simulink model "v01_aa_rii_16r4t11f_ver120sa"  (Simulink 7.6)
settings: Solver=ode45  StartTime=0.0  StopTime=128000  RelTol=1e-3
undefined symbols (not defined in the file or in its folder): sfp
libraries: xbsIndex_r4 x27287, monroe_library x154, xbsReplacements_r4 x78, casper_library_misc x33, simulink x32, dspsigops x32, xps_library x24
(part 5 of 178)

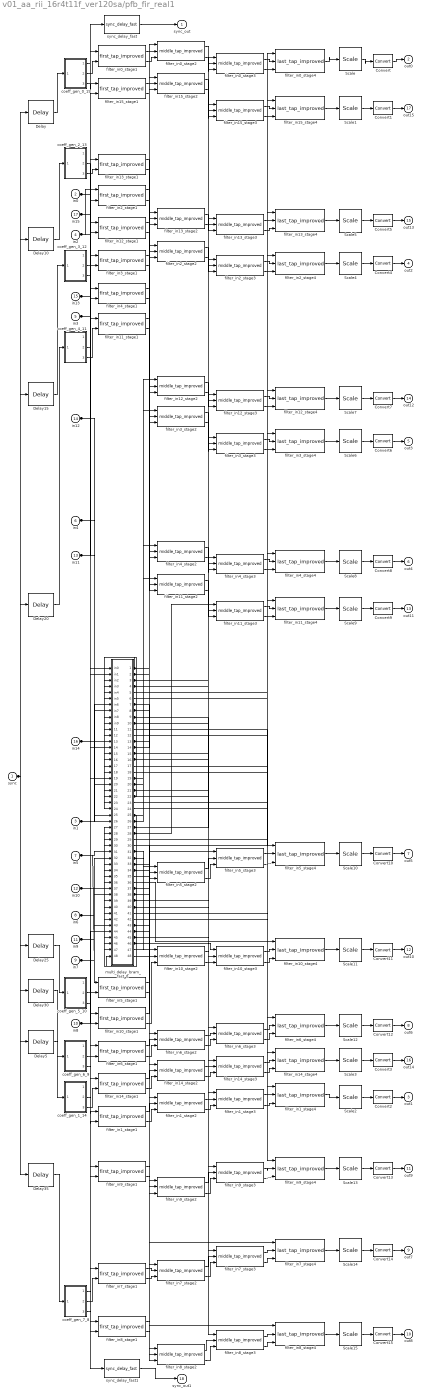
== system v01_aa_rii_16r4t11f_ver120sa/pfb_fir_real1 ==
  sync : Inport
  in0 : Inport  Port=2
  in1 : Inport  Port=3
  in2 : Inport  Port=4
  in3 : Inport  Port=5
  in4 : Inport  Port=6
  in5 : Inport  Port=7
  in6 : Inport  Port=8
  in7 : Inport  Port=9
  in8 : Inport  Port=10
  in9 : Inport  Port=11
  in10 : Inport  Port=12
  in11 : Inport  Port=13
  in12 : Inport  Port=14
  in13 : Inport  Port=15
  in14 : Inport  Port=16
  in15 : Inport  Port=17
  Convert{,1..15} (16 alike) : xbsIndex_r4/Convert  gui_display_data_type=Fixed-point  arith_type="Signed  (2's comp)"  n_bits=18  bin_pt=17  float_type=Single  exp_bits=8  fraction_bits=24  quantization=Truncate  overflow=Wrap  en=off  latency=1  dbl_ovrd=off  pipeline=off  block_type=convert
  Delay{,5,10,15,20,25,30,35} (8 alike) : xbsIndex_r4/Delay  rst=off  infoeditControl=Selection of Reset will increase slice count due to use of real FFs and instead of SRLs  en=off  latency=6  dbl_ovrd=off  reg_retiming=off  block_type=delay
  Scale{,1..15} (16 alike) : xbsIndex_r4/Scale  scale_factor=-1  dbl_ovrd=off  block_type=scale
  coeff_gen_0_15 : Subsystem
  coeff_gen_1_14 : Subsystem
  coeff_gen_2_13 : Subsystem
  coeff_gen_3_12 : Subsystem
  coeff_gen_4_11 : Subsystem
  coeff_gen_5_10 : Subsystem
  coeff_gen_6_9 : Subsystem
  coeff_gen_7_8 : Subsystem
  filter_in0_stage1 : monroe_library/first_tap_improved  SystemSampleTime=-1  data_bin_pt=7  coeff_bit_width=18
  filter_in0_stage2 : monroe_library/middle_tap_improved  SystemSampleTime=-1  data_bin_pt=7  coeff_bit_width=18  stage_num=2
  filter_in0_stage3 : monroe_library/middle_tap_improved  SystemSampleTime=-1  data_bin_pt=7  coeff_bit_width=18  stage_num=3
  filter_in0_stage4 : monroe_library/last_tap_improved  SystemSampleTime=-1  data_bin_pt=7  coeff_bin_pt=17  stage_num=4
  filter_in10_stage1 : monroe_library/first_tap_improved  SystemSampleTime=-1  data_bin_pt=7  coeff_bit_width=18
  filter_in10_stage2 : monroe_library/middle_tap_improved  SystemSampleTime=-1  data_bin_pt=7  coeff_bit_width=18  stage_num=2
  filter_in10_stage3 : monroe_library/middle_tap_improved  SystemSampleTime=-1  data_bin_pt=7  coeff_bit_width=18  stage_num=3
  filter_in10_stage4 : monroe_library/last_tap_improved  SystemSampleTime=-1  data_bin_pt=7  coeff_bin_pt=17  stage_num=4
  filter_in11_stage1 : monroe_library/first_tap_improved  SystemSampleTime=-1  data_bin_pt=7  coeff_bit_width=18
  filter_in11_stage2 : monroe_library/middle_tap_improved  SystemSampleTime=-1  data_bin_pt=7  coeff_bit_width=18  stage_num=2
  filter_in11_stage3 : monroe_library/middle_tap_improved  SystemSampleTime=-1  data_bin_pt=7  coeff_bit_width=18  stage_num=3
  filter_in11_stage4 : monroe_library/last_tap_improved  SystemSampleTime=-1  data_bin_pt=7  coeff_bin_pt=17  stage_num=4
  filter_in12_stage1 : monroe_library/first_tap_improved  SystemSampleTime=-1  data_bin_pt=7  coeff_bit_width=18
  filter_in12_stage2 : monroe_library/middle_tap_improved  SystemSampleTime=-1  data_bin_pt=7  coeff_bit_width=18  stage_num=2
  filter_in12_stage3 : monroe_library/middle_tap_improved  SystemSampleTime=-1  data_bin_pt=7  coeff_bit_width=18  stage_num=3
  filter_in12_stage4 : monroe_library/last_tap_improved  SystemSampleTime=-1  data_bin_pt=7  coeff_bin_pt=17  stage_num=4
  filter_in13_stage1 : monroe_library/first_tap_improved  SystemSampleTime=-1  data_bin_pt=7  coeff_bit_width=18
  filter_in13_stage2 : monroe_library/middle_tap_improved  SystemSampleTime=-1  data_bin_pt=7  coeff_bit_width=18  stage_num=2
  filter_in13_stage3 : monroe_library/middle_tap_improved  SystemSampleTime=-1  data_bin_pt=7  coeff_bit_width=18  stage_num=3
  filter_in13_stage4 : monroe_library/last_tap_improved  SystemSampleTime=-1  data_bin_pt=7  coeff_bin_pt=17  stage_num=4
  filter_in14_stage1 : monroe_library/first_tap_improved  SystemSampleTime=-1  data_bin_pt=7  coeff_bit_width=18
  filter_in14_stage2 : monroe_library/middle_tap_improved  SystemSampleTime=-1  data_bin_pt=7  coeff_bit_width=18  stage_num=2
  filter_in14_stage3 : monroe_library/middle_tap_improved  SystemSampleTime=-1  data_bin_pt=7  coeff_bit_width=18  stage_num=3
  filter_in14_stage4 : monroe_library/last_tap_improved  SystemSampleTime=-1  data_bin_pt=7  coeff_bin_pt=17  stage_num=4
  filter_in15_stage1 : monroe_library/first_tap_improved  SystemSampleTime=-1  data_bin_pt=7  coeff_bit_width=18
  filter_in15_stage2 : monroe_library/middle_tap_improved  SystemSampleTime=-1  data_bin_pt=7  coeff_bit_width=18  stage_num=2
  filter_in15_stage3 : monroe_library/middle_tap_improved  SystemSampleTime=-1  data_bin_pt=7  coeff_bit_width=18  stage_num=3
  filter_in15_stage4 : monroe_library/last_tap_improved  SystemSampleTime=-1  data_bin_pt=7  coeff_bin_pt=17  stage_num=4
  filter_in1_stage1 : monroe_library/first_tap_improved  SystemSampleTime=-1  data_bin_pt=7  coeff_bit_width=18
  filter_in1_stage2 : monroe_library/middle_tap_improved  SystemSampleTime=-1  data_bin_pt=7  coeff_bit_width=18  stage_num=2
  filter_in1_stage3 : monroe_library/middle_tap_improved  SystemSampleTime=-1  data_bin_pt=7  coeff_bit_width=18  stage_num=3
  filter_in1_stage4 : monroe_library/last_tap_improved  SystemSampleTime=-1  data_bin_pt=7  coeff_bin_pt=17  stage_num=4
  filter_in2_stage1 : monroe_library/first_tap_improved  SystemSampleTime=-1  data_bin_pt=7  coeff_bit_width=18
  filter_in2_stage2 : monroe_library/middle_tap_improved  SystemSampleTime=-1  data_bin_pt=7  coeff_bit_width=18  stage_num=2
  filter_in2_stage3 : monroe_library/middle_tap_improved  SystemSampleTime=-1  data_bin_pt=7  coeff_bit_width=18  stage_num=3
  filter_in2_stage4 : monroe_library/last_tap_improved  SystemSampleTime=-1  data_bin_pt=7  coeff_bin_pt=17  stage_num=4
  filter_in3_stage1 : monroe_library/first_tap_improved  SystemSampleTime=-1  data_bin_pt=7  coeff_bit_width=18
  filter_in3_stage2 : monroe_library/middle_tap_improved  SystemSampleTime=-1  data_bin_pt=7  coeff_bit_width=18  stage_num=2
  filter_in3_stage3 : monroe_library/middle_tap_improved  SystemSampleTime=-1  data_bin_pt=7  coeff_bit_width=18  stage_num=3
  filter_in3_stage4 : monroe_library/last_tap_improved  SystemSampleTime=-1  data_bin_pt=7  coeff_bin_pt=17  stage_num=4
  filter_in4_stage1 : monroe_library/first_tap_improved  SystemSampleTime=-1  data_bin_pt=7  coeff_bit_width=18
  filter_in4_stage2 : monroe_library/middle_tap_improved  SystemSampleTime=-1  data_bin_pt=7  coeff_bit_width=18  stage_num=2
  filter_in4_stage3 : monroe_library/middle_tap_improved  SystemSampleTime=-1  data_bin_pt=7  coeff_bit_width=18  stage_num=3
  filter_in4_stage4 : monroe_library/last_tap_improved  SystemSampleTime=-1  data_bin_pt=7  coeff_bin_pt=17  stage_num=4
  filter_in5_stage1 : monroe_library/first_tap_improved  SystemSampleTime=-1  data_bin_pt=7  coeff_bit_width=18
  filter_in5_stage2 : monroe_library/middle_tap_improved  SystemSampleTime=-1  data_bin_pt=7  coeff_bit_width=18  stage_num=2
  filter_in5_stage3 : monroe_library/middle_tap_improved  SystemSampleTime=-1  data_bin_pt=7  coeff_bit_width=18  stage_num=3
  filter_in5_stage4 : monroe_library/last_tap_improved  SystemSampleTime=-1  data_bin_pt=7  coeff_bin_pt=17  stage_num=4
  filter_in6_stage1 : monroe_library/first_tap_improved  SystemSampleTime=-1  data_bin_pt=7  coeff_bit_width=18
  filter_in6_stage2 : monroe_library/middle_tap_improved  SystemSampleTime=-1  data_bin_pt=7  coeff_bit_width=18  stage_num=2
  filter_in6_stage3 : monroe_library/middle_tap_improved  SystemSampleTime=-1  data_bin_pt=7  coeff_bit_width=18  stage_num=3
  filter_in6_stage4 : monroe_library/last_tap_improved  SystemSampleTime=-1  data_bin_pt=7  coeff_bin_pt=17  stage_num=4
  filter_in7_stage1 : monroe_library/first_tap_improved  SystemSampleTime=-1  data_bin_pt=7  coeff_bit_width=18
  filter_in7_stage2 : monroe_library/middle_tap_improved  SystemSampleTime=-1  data_bin_pt=7  coeff_bit_width=18  stage_num=2
  filter_in7_stage3 : monroe_library/middle_tap_improved  SystemSampleTime=-1  data_bin_pt=7  coeff_bit_width=18  stage_num=3
  filter_in7_stage4 : monroe_library/last_tap_improved  SystemSampleTime=-1  data_bin_pt=7  coeff_bin_pt=17  stage_num=4
  filter_in8_stage1 : monroe_library/first_tap_improved  SystemSampleTime=-1  data_bin_pt=7  coeff_bit_width=18
  filter_in8_stage2 : monroe_library/middle_tap_improved  SystemSampleTime=-1  data_bin_pt=7  coeff_bit_width=18  stage_num=2
  filter_in8_stage3 : monroe_library/middle_tap_improved  SystemSampleTime=-1  data_bin_pt=7  coeff_bit_width=18  stage_num=3
  filter_in8_stage4 : monroe_library/last_tap_improved  SystemSampleTime=-1  data_bin_pt=7  coeff_bin_pt=17  stage_num=4
  filter_in9_stage1 : monroe_library/first_tap_improved  SystemSampleTime=-1  data_bin_pt=7  coeff_bit_width=18
  filter_in9_stage2 : monroe_library/middle_tap_improved  SystemSampleTime=-1  data_bin_pt=7  coeff_bit_width=18  stage_num=2
  filter_in9_stage3 : monroe_library/middle_tap_improved  SystemSampleTime=-1  data_bin_pt=7  coeff_bit_width=18  stage_num=3
  filter_in9_stage4 : monroe_library/last_tap_improved  SystemSampleTime=-1  data_bin_pt=7  coeff_bin_pt=17  stage_num=4
  multi_delay_bram_fast_0 : Subsystem
  sync_delay_fast : monroe_library/sync_delay_fast  SystemSampleTime=-1  delay_len=385
  sync_delay_fast1 : monroe_library/sync_delay_fast  SystemSampleTime=-1  delay_len=385
  sync_out : Outport
  out0 : Outport  Port=2
  out1 : Outport  Port=3
  out2 : Outport  Port=4
  out3 : Outport  Port=5
  out4 : Outport  Port=6
  out5 : Outport  Port=7
  out6 : Outport  Port=8
  out7 : Outport  Port=9
  out8 : Outport  Port=10
  out9 : Outport  Port=11
  out10 : Outport  Port=12
  out11 : Outport  Port=13
  out12 : Outport  Port=14
  out13 : Outport  Port=15
  out14 : Outport  Port=16
  out15 : Outport  Port=17
  sync_out1 : Outport  Port=18
  wires:
  sync/1 -> Delay35/1, Delay30/1, Delay25/1, Delay20/1, Delay15/1, Delay10/1, Delay5/1, Delay/1
  in0/1 -> multi_delay_bram_fast_0/1(in0), filter_in0_stage1/1
  in15/1 -> multi_delay_bram_fast_0/2(in1), filter_in15_stage1/1
  in1/1 -> filter_in1_stage1/1, multi_delay_bram_fast_0/7(in6)
  in14/1 -> filter_in14_stage1/1, multi_delay_bram_fast_0/8(in7)
  in2/1 -> filter_in2_stage1/1, multi_delay_bram_fast_0/13(in12)
  in13/1 -> filter_in13_stage1/1, multi_delay_bram_fast_0/14(in13)
  in3/1 -> filter_in3_stage1/1, multi_delay_bram_fast_0/19(in18)
  in12/1 -> filter_in12_stage1/1, multi_delay_bram_fast_0/20(in19)
  in4/1 -> filter_in4_stage1/1, multi_delay_bram_fast_0/25(in24)
  in11/1 -> filter_in11_stage1/1, multi_delay_bram_fast_0/26(in25)
  in5/1 -> filter_in5_stage1/1, multi_delay_bram_fast_0/31(in30)
  in10/1 -> filter_in10_stage1/1, multi_delay_bram_fast_0/32(in31)
  in6/1 -> filter_in6_stage1/1, multi_delay_bram_fast_0/37(in36)
  in9/1 -> filter_in9_stage1/1, multi_delay_bram_fast_0/38(in37)
  in7/1 -> filter_in7_stage1/1, multi_delay_bram_fast_0/43(in42)
  in8/1 -> filter_in8_stage1/1, multi_delay_bram_fast_0/44(in43)
  multi_delay_bram_fast_0/1(out0) -> filter_in0_stage2/1, multi_delay_bram_fast_0/3(in2)
  multi_delay_bram_fast_0/2(out1) -> filter_in15_stage2/1, multi_delay_bram_fast_0/4(in3)
  multi_delay_bram_fast_0/3(out2) -> filter_in0_stage3/1, multi_delay_bram_fast_0/5(in4)
  multi_delay_bram_fast_0/4(out3) -> filter_in15_stage3/1, multi_delay_bram_fast_0/6(in5)
  multi_delay_bram_fast_0/7(out6) -> filter_in1_stage2/1, multi_delay_bram_fast_0/9(in8)
  multi_delay_bram_fast_0/8(out7) -> filter_in14_stage2/1, multi_delay_bram_fast_0/10(in9)
  multi_delay_bram_fast_0/9(out8) -> filter_in1_stage3/1, multi_delay_bram_fast_0/11(in10)
  multi_delay_bram_fast_0/10(out9) -> filter_in14_stage3/1, multi_delay_bram_fast_0/12(in11)
  multi_delay_bram_fast_0/13(out12) -> filter_in2_stage2/1, multi_delay_bram_fast_0/15(in14)
  multi_delay_bram_fast_0/14(out13) -> filter_in13_stage2/1, multi_delay_bram_fast_0/16(in15)
  multi_delay_bram_fast_0/15(out14) -> filter_in2_stage3/1, multi_delay_bram_fast_0/17(in16)
  multi_delay_bram_fast_0/16(out15) -> filter_in13_stage3/1, multi_delay_bram_fast_0/18(in17)
  multi_delay_bram_fast_0/19(out18) -> filter_in3_stage2/1, multi_delay_bram_fast_0/21(in20)
  multi_delay_bram_fast_0/20(out19) -> filter_in12_stage2/1, multi_delay_bram_fast_0/22(in21)
  multi_delay_bram_fast_0/21(out20) -> filter_in3_stage3/1, multi_delay_bram_fast_0/23(in22)
  multi_delay_bram_fast_0/22(out21) -> filter_in12_stage3/1, multi_delay_bram_fast_0/24(in23)
  multi_delay_bram_fast_0/25(out24) -> filter_in4_stage2/1, multi_delay_bram_fast_0/27(in26)
  multi_delay_bram_fast_0/26(out25) -> filter_in11_stage2/1, multi_delay_bram_fast_0/28(in27)
  multi_delay_bram_fast_0/27(out26) -> filter_in4_stage3/1, multi_delay_bram_fast_0/29(in28)
  multi_delay_bram_fast_0/28(out27) -> filter_in11_stage3/1, multi_delay_bram_fast_0/30(in29)
  multi_delay_bram_fast_0/31(out30) -> filter_in5_stage2/1, multi_delay_bram_fast_0/33(in32)
  multi_delay_bram_fast_0/32(out31) -> filter_in10_stage2/1, multi_delay_bram_fast_0/34(in33)
  multi_delay_bram_fast_0/33(out32) -> filter_in5_stage3/1, multi_delay_bram_fast_0/35(in34)
  multi_delay_bram_fast_0/34(out33) -> filter_in10_stage3/1, multi_delay_bram_fast_0/36(in35)
  multi_delay_bram_fast_0/37(out36) -> filter_in6_stage2/1, multi_delay_bram_fast_0/39(in38)
  multi_delay_bram_fast_0/38(out37) -> filter_in9_stage2/1, multi_delay_bram_fast_0/40(in39)
  multi_delay_bram_fast_0/39(out38) -> filter_in6_stage3/1, multi_delay_bram_fast_0/41(in40)
  multi_delay_bram_fast_0/40(out39) -> filter_in9_stage3/1, multi_delay_bram_fast_0/42(in41)
  multi_delay_bram_fast_0/43(out42) -> filter_in7_stage2/1, multi_delay_bram_fast_0/45(in44)
  multi_delay_bram_fast_0/44(out43) -> filter_in8_stage2/1, multi_delay_bram_fast_0/46(in45)
  multi_delay_bram_fast_0/45(out44) -> filter_in7_stage3/1, multi_delay_bram_fast_0/47(in46)
  multi_delay_bram_fast_0/46(out45) -> filter_in8_stage3/1, multi_delay_bram_fast_0/48(in47)
  sync_delay_fast1/1 -> sync_out1/1
  coeff_gen_7_8/1(sync_out) -> sync_delay_fast1/1
  sync_delay_fast/1 -> sync_out/1
  coeff_gen_0_15/1(sync_out) -> sync_delay_fast/1
  Convert15/1 -> out8/1
  Scale15/1 -> Convert15/1
  Convert14/1 -> out7/1
  Scale14/1 -> Convert14/1
  filter_in8_stage4/1 -> Scale15/1
  filter_in7_stage4/1 -> Scale14/1
  filter_in8_stage3/2 -> filter_in8_stage4/3
  filter_in8_stage3/1 -> filter_in8_stage4/2
  multi_delay_bram_fast_0/48(out47) -> filter_in8_stage4/1
  filter_in7_stage3/2 -> filter_in7_stage4/3
  filter_in7_stage3/1 -> filter_in7_stage4/2
  multi_delay_bram_fast_0/47(out46) -> filter_in7_stage4/1
  filter_in8_stage2/2 -> filter_in8_stage3/3
  filter_in8_stage2/1 -> filter_in8_stage3/2
  filter_in7_stage2/2 -> filter_in7_stage3/3
  filter_in7_stage2/1 -> filter_in7_stage3/2
  filter_in8_stage1/2 -> filter_in8_stage2/3
  filter_in8_stage1/1 -> filter_in8_stage2/2
  filter_in7_stage1/2 -> filter_in7_stage2/3
  filter_in7_stage1/1 -> filter_in7_stage2/2
  coeff_gen_7_8/3(coeff_rev) -> filter_in8_stage1/2
  coeff_gen_7_8/2(coeff) -> filter_in7_stage1/2
  Delay35/1 -> coeff_gen_7_8/1(sync)
  Convert13/1 -> out9/1
  Scale13/1 -> Convert13/1
  Convert12/1 -> out6/1
  Scale12/1 -> Convert12/1
  filter_in9_stage4/1 -> Scale13/1
  filter_in6_stage4/1 -> Scale12/1
  filter_in9_stage3/2 -> filter_in9_stage4/3
  filter_in9_stage3/1 -> filter_in9_stage4/2
  multi_delay_bram_fast_0/42(out41) -> filter_in9_stage4/1
  filter_in6_stage3/2 -> filter_in6_stage4/3
  filter_in6_stage3/1 -> filter_in6_stage4/2
  multi_delay_bram_fast_0/41(out40) -> filter_in6_stage4/1
  filter_in9_stage2/2 -> filter_in9_stage3/3
  filter_in9_stage2/1 -> filter_in9_stage3/2
  filter_in6_stage2/2 -> filter_in6_stage3/3
  filter_in6_stage2/1 -> filter_in6_stage3/2
  filter_in9_stage1/2 -> filter_in9_stage2/3
  filter_in9_stage1/1 -> filter_in9_stage2/2
  filter_in6_stage1/2 -> filter_in6_stage2/3
  filter_in6_stage1/1 -> filter_in6_stage2/2
  coeff_gen_6_9/3(coeff_rev) -> filter_in9_stage1/2
  coeff_gen_6_9/2(coeff) -> filter_in6_stage1/2
  Delay30/1 -> coeff_gen_6_9/1(sync)
  Convert11/1 -> out10/1
  Scale11/1 -> Convert11/1
  Convert10/1 -> out5/1
  Scale10/1 -> Convert10/1
  filter_in10_stage4/1 -> Scale11/1
  filter_in5_stage4/1 -> Scale10/1
  filter_in10_stage3/2 -> filter_in10_stage4/3
  filter_in10_stage3/1 -> filter_in10_stage4/2
  multi_delay_bram_fast_0/36(out35) -> filter_in10_stage4/1
  filter_in5_stage3/2 -> filter_in5_stage4/3
  filter_in5_stage3/1 -> filter_in5_stage4/2
  multi_delay_bram_fast_0/35(out34) -> filter_in5_stage4/1
  filter_in10_stage2/2 -> filter_in10_stage3/3
  filter_in10_stage2/1 -> filter_in10_stage3/2
  filter_in5_stage2/2 -> filter_in5_stage3/3
  filter_in5_stage2/1 -> filter_in5_stage3/2
  filter_in10_stage1/2 -> filter_in10_stage2/3
  filter_in10_stage1/1 -> filter_in10_stage2/2
  filter_in5_stage1/2 -> filter_in5_stage2/3
  filter_in5_stage1/1 -> filter_in5_stage2/2
  coeff_gen_5_10/3(coeff_rev) -> filter_in10_stage1/2
  coeff_gen_5_10/2(coeff) -> filter_in5_stage1/2
  Delay25/1 -> coeff_gen_5_10/1(sync)
  Convert9/1 -> out11/1
  Scale9/1 -> Convert9/1
  Convert8/1 -> out4/1
  Scale8/1 -> Convert8/1
  filter_in11_stage4/1 -> Scale9/1
  filter_in4_stage4/1 -> Scale8/1
  filter_in11_stage3/2 -> filter_in11_stage4/3
  filter_in11_stage3/1 -> filter_in11_stage4/2
  multi_delay_bram_fast_0/30(out29) -> filter_in11_stage4/1
  filter_in4_stage3/2 -> filter_in4_stage4/3
  filter_in4_stage3/1 -> filter_in4_stage4/2
  multi_delay_bram_fast_0/29(out28) -> filter_in4_stage4/1
  filter_in11_stage2/2 -> filter_in11_stage3/3
  filter_in11_stage2/1 -> filter_in11_stage3/2
  filter_in4_stage2/2 -> filter_in4_stage3/3
  filter_in4_stage2/1 -> filter_in4_stage3/2
  filter_in11_stage1/2 -> filter_in11_stage2/3
  filter_in11_stage1/1 -> filter_in11_stage2/2
  filter_in4_stage1/2 -> filter_in4_stage2/3
  filter_in4_stage1/1 -> filter_in4_stage2/2
  coeff_gen_4_11/3(coeff_rev) -> filter_in11_stage1/2
  coeff_gen_4_11/2(coeff) -> filter_in4_stage1/2
  Delay20/1 -> coeff_gen_4_11/1(sync)
  Convert7/1 -> out12/1
  Scale7/1 -> Convert7/1
  Convert6/1 -> out3/1
  Scale6/1 -> Convert6/1
  filter_in12_stage4/1 -> Scale7/1
  filter_in3_stage4/1 -> Scale6/1
  filter_in12_stage3/2 -> filter_in12_stage4/3
  filter_in12_stage3/1 -> filter_in12_stage4/2
  multi_delay_bram_fast_0/24(out23) -> filter_in12_stage4/1
  filter_in3_stage3/2 -> filter_in3_stage4/3
  filter_in3_stage3/1 -> filter_in3_stage4/2
  multi_delay_bram_fast_0/23(out22) -> filter_in3_stage4/1
  filter_in12_stage2/2 -> filter_in12_stage3/3
  filter_in12_stage2/1 -> filter_in12_stage3/2
  filter_in3_stage2/2 -> filter_in3_stage3/3
  filter_in3_stage2/1 -> filter_in3_stage3/2
  filter_in12_stage1/2 -> filter_in12_stage2/3
  filter_in12_stage1/1 -> filter_in12_stage2/2
  filter_in3_stage1/2 -> filter_in3_stage2/3
  filter_in3_stage1/1 -> filter_in3_stage2/2
  coeff_gen_3_12/3(coeff_rev) -> filter_in12_stage1/2
  coeff_gen_3_12/2(coeff) -> filter_in3_stage1/2
  Delay15/1 -> coeff_gen_3_12/1(sync)
  Convert5/1 -> out13/1
  Scale5/1 -> Convert5/1
  Convert4/1 -> out2/1
  Scale4/1 -> Convert4/1
  filter_in13_stage4/1 -> Scale5/1
  filter_in2_stage4/1 -> Scale4/1
  filter_in13_stage3/2 -> filter_in13_stage4/3
  filter_in13_stage3/1 -> filter_in13_stage4/2
  multi_delay_bram_fast_0/18(out17) -> filter_in13_stage4/1
  filter_in2_stage3/2 -> filter_in2_stage4/3
  filter_in2_stage3/1 -> filter_in2_stage4/2
  multi_delay_bram_fast_0/17(out16) -> filter_in2_stage4/1
  filter_in13_stage2/2 -> filter_in13_stage3/3
  filter_in13_stage2/1 -> filter_in13_stage3/2
  filter_in2_stage2/2 -> filter_in2_stage3/3
  filter_in2_stage2/1 -> filter_in2_stage3/2
  filter_in13_stage1/2 -> filter_in13_stage2/3
  filter_in13_stage1/1 -> filter_in13_stage2/2
  filter_in2_stage1/2 -> filter_in2_stage2/3
  filter_in2_stage1/1 -> filter_in2_stage2/2
  coeff_gen_2_13/3(coeff_rev) -> filter_in13_stage1/2
  coeff_gen_2_13/2(coeff) -> filter_in2_stage1/2
  Delay10/1 -> coeff_gen_2_13/1(sync)
  Convert3/1 -> out14/1
  Scale3/1 -> Convert3/1
  Convert2/1 -> out1/1
  Scale2/1 -> Convert2/1
  filter_in14_stage4/1 -> Scale3/1
  filter_in1_stage4/1 -> Scale2/1
  filter_in14_stage3/2 -> filter_in14_stage4/3
  filter_in14_stage3/1 -> filter_in14_stage4/2
  multi_delay_bram_fast_0/12(out11) -> filter_in14_stage4/1
  filter_in1_stage3/2 -> filter_in1_stage4/3
  filter_in1_stage3/1 -> filter_in1_stage4/2
  multi_delay_bram_fast_0/11(out10) -> filter_in1_stage4/1
  filter_in14_stage2/2 -> filter_in14_stage3/3
  filter_in14_stage2/1 -> filter_in14_stage3/2
  filter_in1_stage2/2 -> filter_in1_stage3/3
  filter_in1_stage2/1 -> filter_in1_stage3/2
  filter_in14_stage1/2 -> filter_in14_stage2/3
  filter_in14_stage1/1 -> filter_in14_stage2/2
  filter_in1_stage1/2 -> filter_in1_stage2/3
  filter_in1_stage1/1 -> filter_in1_stage2/2
  coeff_gen_1_14/3(coeff_rev) -> filter_in14_stage1/2
  coeff_gen_1_14/2(coeff) -> filter_in1_stage1/2
  Delay5/1 -> coeff_gen_1_14/1(sync)
  Convert1/1 -> out15/1
  Scale1/1 -> Convert1/1
  Convert/1 -> out0/1
  Scale/1 -> Convert/1
  filter_in15_stage4/1 -> Scale1/1
  filter_in0_stage4/1 -> Scale/1
  filter_in15_stage3/2 -> filter_in15_stage4/3
  filter_in15_stage3/1 -> filter_in15_stage4/2
  multi_delay_bram_fast_0/6(out5) -> filter_in15_stage4/1
  filter_in0_stage3/2 -> filter_in0_stage4/3
  filter_in0_stage3/1 -> filter_in0_stage4/2
  multi_delay_bram_fast_0/5(out4) -> filter_in0_stage4/1
  filter_in15_stage2/2 -> filter_in15_stage3/3
  filter_in15_stage2/1 -> filter_in15_stage3/2
  filter_in0_stage2/2 -> filter_in0_stage3/3
  filter_in0_stage2/1 -> filter_in0_stage3/2
  filter_in15_stage1/2 -> filter_in15_stage2/3
  filter_in15_stage1/1 -> filter_in15_stage2/2
  filter_in0_stage1/2 -> filter_in0_stage2/3
  filter_in0_stage1/1 -> filter_in0_stage2/2
  coeff_gen_0_15/3(coeff_rev) -> filter_in15_stage1/2
  coeff_gen_0_15/2(coeff) -> filter_in0_stage1/2
  Delay/1 -> coeff_gen_0_15/1(sync)

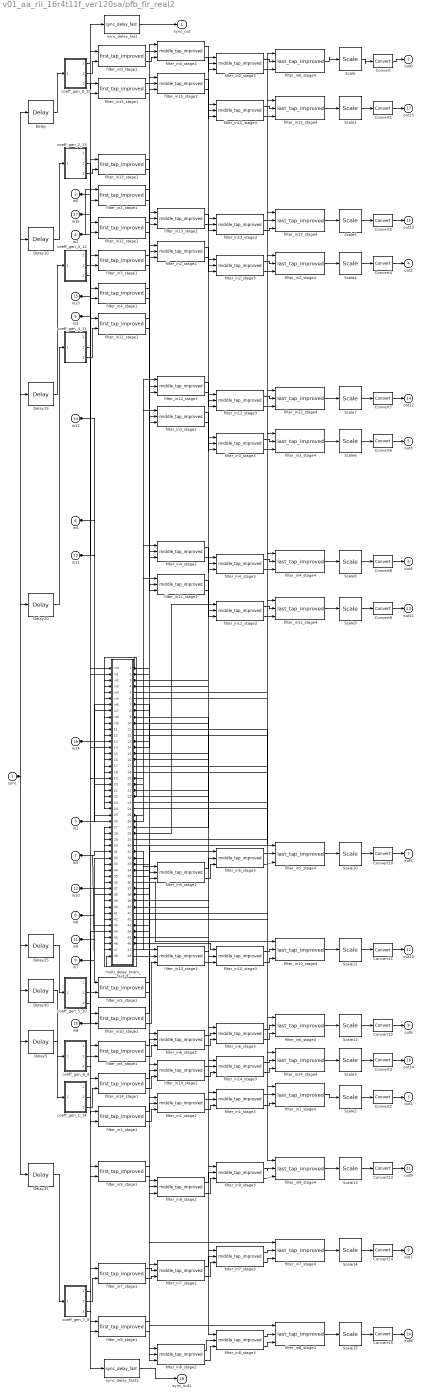
== system v01_aa_rii_16r4t11f_ver120sa/pfb_fir_real2 ==
  sync : Inport
  in0 : Inport  Port=2
  in1 : Inport  Port=3
  in2 : Inport  Port=4
  in3 : Inport  Port=5
  in4 : Inport  Port=6
  in5 : Inport  Port=7
  in6 : Inport  Port=8
  in7 : Inport  Port=9
  in8 : Inport  Port=10
  in9 : Inport  Port=11
  in10 : Inport  Port=12
  in11 : Inport  Port=13
  in12 : Inport  Port=14
  in13 : Inport  Port=15
  in14 : Inport  Port=16
  in15 : Inport  Port=17
  Convert{,1..15} (16 alike) : xbsIndex_r4/Convert  gui_display_data_type=Fixed-point  arith_type="Signed  (2's comp)"  n_bits=18  bin_pt=17  float_type=Single  exp_bits=8  fraction_bits=24  quantization=Truncate  overflow=Wrap  en=off  latency=1  dbl_ovrd=off  pipeline=off  block_type=convert
  Delay{,5,10,15,20,25,30,35} (8 alike) : xbsIndex_r4/Delay  rst=off  infoeditControl=Selection of Reset will increase slice count due to use of real FFs and instead of SRLs  en=off  latency=6  dbl_ovrd=off  reg_retiming=off  block_type=delay
  Scale{,1..15} (16 alike) : xbsIndex_r4/Scale  scale_factor=-1  dbl_ovrd=off  block_type=scale
  coeff_gen_0_15 : Subsystem
  coeff_gen_1_14 : Subsystem
  coeff_gen_2_13 : Subsystem
  coeff_gen_3_12 : Subsystem
  coeff_gen_4_11 : Subsystem
  coeff_gen_5_10 : Subsystem
  coeff_gen_6_9 : Subsystem
  coeff_gen_7_8 : Subsystem
  filter_in0_stage1 : monroe_library/first_tap_improved  SystemSampleTime=-1  data_bin_pt=7  coeff_bit_width=18
  filter_in0_stage2 : monroe_library/middle_tap_improved  SystemSampleTime=-1  data_bin_pt=7  coeff_bit_width=18  stage_num=2
  filter_in0_stage3 : monroe_library/middle_tap_improved  SystemSampleTime=-1  data_bin_pt=7  coeff_bit_width=18  stage_num=3
  filter_in0_stage4 : monroe_library/last_tap_improved  SystemSampleTime=-1  data_bin_pt=7  coeff_bin_pt=17  stage_num=4
  filter_in10_stage1 : monroe_library/first_tap_improved  SystemSampleTime=-1  data_bin_pt=7  coeff_bit_width=18
  filter_in10_stage2 : monroe_library/middle_tap_improved  SystemSampleTime=-1  data_bin_pt=7  coeff_bit_width=18  stage_num=2
  filter_in10_stage3 : monroe_library/middle_tap_improved  SystemSampleTime=-1  data_bin_pt=7  coeff_bit_width=18  stage_num=3
  filter_in10_stage4 : monroe_library/last_tap_improved  SystemSampleTime=-1  data_bin_pt=7  coeff_bin_pt=17  stage_num=4
  filter_in11_stage1 : monroe_library/first_tap_improved  SystemSampleTime=-1  data_bin_pt=7  coeff_bit_width=18
  filter_in11_stage2 : monroe_library/middle_tap_improved  SystemSampleTime=-1  data_bin_pt=7  coeff_bit_width=18  stage_num=2
  filter_in11_stage3 : monroe_library/middle_tap_improved  SystemSampleTime=-1  data_bin_pt=7  coeff_bit_width=18  stage_num=3
  filter_in11_stage4 : monroe_library/last_tap_improved  SystemSampleTime=-1  data_bin_pt=7  coeff_bin_pt=17  stage_num=4
  filter_in12_stage1 : monroe_library/first_tap_improved  SystemSampleTime=-1  data_bin_pt=7  coeff_bit_width=18
  filter_in12_stage2 : monroe_library/middle_tap_improved  SystemSampleTime=-1  data_bin_pt=7  coeff_bit_width=18  stage_num=2
  filter_in12_stage3 : monroe_library/middle_tap_improved  SystemSampleTime=-1  data_bin_pt=7  coeff_bit_width=18  stage_num=3
  filter_in12_stage4 : monroe_library/last_tap_improved  SystemSampleTime=-1  data_bin_pt=7  coeff_bin_pt=17  stage_num=4
  filter_in13_stage1 : monroe_library/first_tap_improved  SystemSampleTime=-1  data_bin_pt=7  coeff_bit_width=18
  filter_in13_stage2 : monroe_library/middle_tap_improved  SystemSampleTime=-1  data_bin_pt=7  coeff_bit_width=18  stage_num=2
  filter_in13_stage3 : monroe_library/middle_tap_improved  SystemSampleTime=-1  data_bin_pt=7  coeff_bit_width=18  stage_num=3
  filter_in13_stage4 : monroe_library/last_tap_improved  SystemSampleTime=-1  data_bin_pt=7  coeff_bin_pt=17  stage_num=4
  filter_in14_stage1 : monroe_library/first_tap_improved  SystemSampleTime=-1  data_bin_pt=7  coeff_bit_width=18
  filter_in14_stage2 : monroe_library/middle_tap_improved  SystemSampleTime=-1  data_bin_pt=7  coeff_bit_width=18  stage_num=2
  filter_in14_stage3 : monroe_library/middle_tap_improved  SystemSampleTime=-1  data_bin_pt=7  coeff_bit_width=18  stage_num=3
  filter_in14_stage4 : monroe_library/last_tap_improved  SystemSampleTime=-1  data_bin_pt=7  coeff_bin_pt=17  stage_num=4
  filter_in15_stage1 : monroe_library/first_tap_improved  SystemSampleTime=-1  data_bin_pt=7  coeff_bit_width=18
  filter_in15_stage2 : monroe_library/middle_tap_improved  SystemSampleTime=-1  data_bin_pt=7  coeff_bit_width=18  stage_num=2
  filter_in15_stage3 : monroe_library/middle_tap_improved  SystemSampleTime=-1  data_bin_pt=7  coeff_bit_width=18  stage_num=3
  filter_in15_stage4 : monroe_library/last_tap_improved  SystemSampleTime=-1  data_bin_pt=7  coeff_bin_pt=17  stage_num=4
  filter_in1_stage1 : monroe_library/first_tap_improved  SystemSampleTime=-1  data_bin_pt=7  coeff_bit_width=18
  filter_in1_stage2 : monroe_library/middle_tap_improved  SystemSampleTime=-1  data_bin_pt=7  coeff_bit_width=18  stage_num=2
  filter_in1_stage3 : monroe_library/middle_tap_improved  SystemSampleTime=-1  data_bin_pt=7  coeff_bit_width=18  stage_num=3
  filter_in1_stage4 : monroe_library/last_tap_improved  SystemSampleTime=-1  data_bin_pt=7  coeff_bin_pt=17  stage_num=4
  filter_in2_stage1 : monroe_library/first_tap_improved  SystemSampleTime=-1  data_bin_pt=7  coeff_bit_width=18
  filter_in2_stage2 : monroe_library/middle_tap_improved  SystemSampleTime=-1  data_bin_pt=7  coeff_bit_width=18  stage_num=2
  filter_in2_stage3 : monroe_library/middle_tap_improved  SystemSampleTime=-1  data_bin_pt=7  coeff_bit_width=18  stage_num=3
  filter_in2_stage4 : monroe_library/last_tap_improved  SystemSampleTime=-1  data_bin_pt=7  coeff_bin_pt=17  stage_num=4
  filter_in3_stage1 : monroe_library/first_tap_improved  SystemSampleTime=-1  data_bin_pt=7  coeff_bit_width=18
  filter_in3_stage2 : monroe_library/middle_tap_improved  SystemSampleTime=-1  data_bin_pt=7  coeff_bit_width=18  stage_num=2
  filter_in3_stage3 : monroe_library/middle_tap_improved  SystemSampleTime=-1  data_bin_pt=7  coeff_bit_width=18  stage_num=3
  filter_in3_stage4 : monroe_library/last_tap_improved  SystemSampleTime=-1  data_bin_pt=7  coeff_bin_pt=17  stage_num=4
  filter_in4_stage1 : monroe_library/first_tap_improved  SystemSampleTime=-1  data_bin_pt=7  coeff_bit_width=18
  filter_in4_stage2 : monroe_library/middle_tap_improved  SystemSampleTime=-1  data_bin_pt=7  coeff_bit_width=18  stage_num=2
  filter_in4_stage3 : monroe_library/middle_tap_improved  SystemSampleTime=-1  data_bin_pt=7  coeff_bit_width=18  stage_num=3
  filter_in4_stage4 : monroe_library/last_tap_improved  SystemSampleTime=-1  data_bin_pt=7  coeff_bin_pt=17  stage_num=4
  filter_in5_stage1 : monroe_library/first_tap_improved  SystemSampleTime=-1  data_bin_pt=7  coeff_bit_width=18
  filter_in5_stage2 : monroe_library/middle_tap_improved  SystemSampleTime=-1  data_bin_pt=7  coeff_bit_width=18  stage_num=2
  filter_in5_stage3 : monroe_library/middle_tap_improved  SystemSampleTime=-1  data_bin_pt=7  coeff_bit_width=18  stage_num=3
  filter_in5_stage4 : monroe_library/last_tap_improved  SystemSampleTime=-1  data_bin_pt=7  coeff_bin_pt=17  stage_num=4
  filter_in6_stage1 : monroe_library/first_tap_improved  SystemSampleTime=-1  data_bin_pt=7  coeff_bit_width=18
  filter_in6_stage2 : monroe_library/middle_tap_improved  SystemSampleTime=-1  data_bin_pt=7  coeff_bit_width=18  stage_num=2
  filter_in6_stage3 : monroe_library/middle_tap_improved  SystemSampleTime=-1  data_bin_pt=7  coeff_bit_width=18  stage_num=3
  filter_in6_stage4 : monroe_library/last_tap_improved  SystemSampleTime=-1  data_bin_pt=7  coeff_bin_pt=17  stage_num=4
  filter_in7_stage1 : monroe_library/first_tap_improved  SystemSampleTime=-1  data_bin_pt=7  coeff_bit_width=18
  filter_in7_stage2 : monroe_library/middle_tap_improved  SystemSampleTime=-1  data_bin_pt=7  coeff_bit_width=18  stage_num=2
  filter_in7_stage3 : monroe_library/middle_tap_improved  SystemSampleTime=-1  data_bin_pt=7  coeff_bit_width=18  stage_num=3
  filter_in7_stage4 : monroe_library/last_tap_improved  SystemSampleTime=-1  data_bin_pt=7  coeff_bin_pt=17  stage_num=4
  filter_in8_stage1 : monroe_library/first_tap_improved  SystemSampleTime=-1  data_bin_pt=7  coeff_bit_width=18
  filter_in8_stage2 : monroe_library/middle_tap_improved  SystemSampleTime=-1  data_bin_pt=7  coeff_bit_width=18  stage_num=2
  filter_in8_stage3 : monroe_library/middle_tap_improved  SystemSampleTime=-1  data_bin_pt=7  coeff_bit_width=18  stage_num=3
  filter_in8_stage4 : monroe_library/last_tap_improved  SystemSampleTime=-1  data_bin_pt=7  coeff_bin_pt=17  stage_num=4
  filter_in9_stage1 : monroe_library/first_tap_improved  SystemSampleTime=-1  data_bin_pt=7  coeff_bit_width=18
  filter_in9_stage2 : monroe_library/middle_tap_improved  SystemSampleTime=-1  data_bin_pt=7  coeff_bit_width=18  stage_num=2
  filter_in9_stage3 : monroe_library/middle_tap_improved  SystemSampleTime=-1  data_bin_pt=7  coeff_bit_width=18  stage_num=3
  filter_in9_stage4 : monroe_library/last_tap_improved  SystemSampleTime=-1  data_bin_pt=7  coeff_bin_pt=17  stage_num=4
  multi_delay_bram_fast_0 : Subsystem
  sync_delay_fast : monroe_library/sync_delay_fast  SystemSampleTime=-1  delay_len=385
  sync_delay_fast1 : monroe_library/sync_delay_fast  SystemSampleTime=-1  delay_len=385
  sync_out : Outport
  out0 : Outport  Port=2
  out1 : Outport  Port=3
  out2 : Outport  Port=4
  out3 : Outport  Port=5
  out4 : Outport  Port=6
  out5 : Outport  Port=7
  out6 : Outport  Port=8
  out7 : Outport  Port=9
  out8 : Outport  Port=10
  out9 : Outport  Port=11
  out10 : Outport  Port=12
  out11 : Outport  Port=13
  out12 : Outport  Port=14
  out13 : Outport  Port=15
  out14 : Outport  Port=16
  out15 : Outport  Port=17
  sync_out1 : Outport  Port=18
  wires:
  sync/1 -> Delay35/1, Delay30/1, Delay25/1, Delay20/1, Delay15/1, Delay10/1, Delay5/1, Delay/1
  in0/1 -> multi_delay_bram_fast_0/1(in0), filter_in0_stage1/1
  in15/1 -> multi_delay_bram_fast_0/2(in1), filter_in15_stage1/1
  in1/1 -> filter_in1_stage1/1, multi_delay_bram_fast_0/7(in6)
  in14/1 -> filter_in14_stage1/1, multi_delay_bram_fast_0/8(in7)
  in2/1 -> filter_in2_stage1/1, multi_delay_bram_fast_0/13(in12)
  in13/1 -> filter_in13_stage1/1, multi_delay_bram_fast_0/14(in13)
  in3/1 -> filter_in3_stage1/1, multi_delay_bram_fast_0/19(in18)
  in12/1 -> filter_in12_stage1/1, multi_delay_bram_fast_0/20(in19)
  in4/1 -> filter_in4_stage1/1, multi_delay_bram_fast_0/25(in24)
  in11/1 -> filter_in11_stage1/1, multi_delay_bram_fast_0/26(in25)
  in5/1 -> filter_in5_stage1/1, multi_delay_bram_fast_0/31(in30)
  in10/1 -> filter_in10_stage1/1, multi_delay_bram_fast_0/32(in31)
  in6/1 -> filter_in6_stage1/1, multi_delay_bram_fast_0/37(in36)
  in9/1 -> filter_in9_stage1/1, multi_delay_bram_fast_0/38(in37)
  in7/1 -> filter_in7_stage1/1, multi_delay_bram_fast_0/43(in42)
  in8/1 -> filter_in8_stage1/1, multi_delay_bram_fast_0/44(in43)
  multi_delay_bram_fast_0/1(out0) -> filter_in0_stage2/1, multi_delay_bram_fast_0/3(in2)
  multi_delay_bram_fast_0/2(out1) -> filter_in15_stage2/1, multi_delay_bram_fast_0/4(in3)
  multi_delay_bram_fast_0/3(out2) -> filter_in0_stage3/1, multi_delay_bram_fast_0/5(in4)
  multi_delay_bram_fast_0/4(out3) -> filter_in15_stage3/1, multi_delay_bram_fast_0/6(in5)
  multi_delay_bram_fast_0/7(out6) -> filter_in1_stage2/1, multi_delay_bram_fast_0/9(in8)
  multi_delay_bram_fast_0/8(out7) -> filter_in14_stage2/1, multi_delay_bram_fast_0/10(in9)
  multi_delay_bram_fast_0/9(out8) -> filter_in1_stage3/1, multi_delay_bram_fast_0/11(in10)
  multi_delay_bram_fast_0/10(out9) -> filter_in14_stage3/1, multi_delay_bram_fast_0/12(in11)
  multi_delay_bram_fast_0/13(out12) -> filter_in2_stage2/1, multi_delay_bram_fast_0/15(in14)
  multi_delay_bram_fast_0/14(out13) -> filter_in13_stage2/1, multi_delay_bram_fast_0/16(in15)
  multi_delay_bram_fast_0/15(out14) -> filter_in2_stage3/1, multi_delay_bram_fast_0/17(in16)
  multi_delay_bram_fast_0/16(out15) -> filter_in13_stage3/1, multi_delay_bram_fast_0/18(in17)
  multi_delay_bram_fast_0/19(out18) -> filter_in3_stage2/1, multi_delay_bram_fast_0/21(in20)
  multi_delay_bram_fast_0/20(out19) -> filter_in12_stage2/1, multi_delay_bram_fast_0/22(in21)
  multi_delay_bram_fast_0/21(out20) -> filter_in3_stage3/1, multi_delay_bram_fast_0/23(in22)
  multi_delay_bram_fast_0/22(out21) -> filter_in12_stage3/1, multi_delay_bram_fast_0/24(in23)
  multi_delay_bram_fast_0/25(out24) -> filter_in4_stage2/1, multi_delay_bram_fast_0/27(in26)
  multi_delay_bram_fast_0/26(out25) -> filter_in11_stage2/1, multi_delay_bram_fast_0/28(in27)
  multi_delay_bram_fast_0/27(out26) -> filter_in4_stage3/1, multi_delay_bram_fast_0/29(in28)
  multi_delay_bram_fast_0/28(out27) -> filter_in11_stage3/1, multi_delay_bram_fast_0/30(in29)
  multi_delay_bram_fast_0/31(out30) -> filter_in5_stage2/1, multi_delay_bram_fast_0/33(in32)
  multi_delay_bram_fast_0/32(out31) -> filter_in10_stage2/1, multi_delay_bram_fast_0/34(in33)
  multi_delay_bram_fast_0/33(out32) -> filter_in5_stage3/1, multi_delay_bram_fast_0/35(in34)
  multi_delay_bram_fast_0/34(out33) -> filter_in10_stage3/1, multi_delay_bram_fast_0/36(in35)
  multi_delay_bram_fast_0/37(out36) -> filter_in6_stage2/1, multi_delay_bram_fast_0/39(in38)
  multi_delay_bram_fast_0/38(out37) -> filter_in9_stage2/1, multi_delay_bram_fast_0/40(in39)
  multi_delay_bram_fast_0/39(out38) -> filter_in6_stage3/1, multi_delay_bram_fast_0/41(in40)
  multi_delay_bram_fast_0/40(out39) -> filter_in9_stage3/1, multi_delay_bram_fast_0/42(in41)
  multi_delay_bram_fast_0/43(out42) -> filter_in7_stage2/1, multi_delay_bram_fast_0/45(in44)
  multi_delay_bram_fast_0/44(out43) -> filter_in8_stage2/1, multi_delay_bram_fast_0/46(in45)
  multi_delay_bram_fast_0/45(out44) -> filter_in7_stage3/1, multi_delay_bram_fast_0/47(in46)
  multi_delay_bram_fast_0/46(out45) -> filter_in8_stage3/1, multi_delay_bram_fast_0/48(in47)
  sync_delay_fast1/1 -> sync_out1/1
  coeff_gen_7_8/1(sync_out) -> sync_delay_fast1/1
  sync_delay_fast/1 -> sync_out/1
  coeff_gen_0_15/1(sync_out) -> sync_delay_fast/1
  Convert15/1 -> out8/1
  Scale15/1 -> Convert15/1
  Convert14/1 -> out7/1
  Scale14/1 -> Convert14/1
  filter_in8_stage4/1 -> Scale15/1
  filter_in7_stage4/1 -> Scale14/1
  filter_in8_stage3/2 -> filter_in8_stage4/3
  filter_in8_stage3/1 -> filter_in8_stage4/2
  multi_delay_bram_fast_0/48(out47) -> filter_in8_stage4/1
  filter_in7_stage3/2 -> filter_in7_stage4/3
  filter_in7_stage3/1 -> filter_in7_stage4/2
  multi_delay_bram_fast_0/47(out46) -> filter_in7_stage4/1
  filter_in8_stage2/2 -> filter_in8_stage3/3
  filter_in8_stage2/1 -> filter_in8_stage3/2
  filter_in7_stage2/2 -> filter_in7_stage3/3
  filter_in7_stage2/1 -> filter_in7_stage3/2
  filter_in8_stage1/2 -> filter_in8_stage2/3
  filter_in8_stage1/1 -> filter_in8_stage2/2
  filter_in7_stage1/2 -> filter_in7_stage2/3
  filter_in7_stage1/1 -> filter_in7_stage2/2
  coeff_gen_7_8/3(coeff_rev) -> filter_in8_stage1/2
  coeff_gen_7_8/2(coeff) -> filter_in7_stage1/2
  Delay35/1 -> coeff_gen_7_8/1(sync)
  Convert13/1 -> out9/1
  Scale13/1 -> Convert13/1
  Convert12/1 -> out6/1
  Scale12/1 -> Convert12/1
  filter_in9_stage4/1 -> Scale13/1
  filter_in6_stage4/1 -> Scale12/1
  filter_in9_stage3/2 -> filter_in9_stage4/3
  filter_in9_stage3/1 -> filter_in9_stage4/2
  multi_delay_bram_fast_0/42(out41) -> filter_in9_stage4/1
  filter_in6_stage3/2 -> filter_in6_stage4/3
  filter_in6_stage3/1 -> filter_in6_stage4/2
  multi_delay_bram_fast_0/41(out40) -> filter_in6_stage4/1
  filter_in9_stage2/2 -> filter_in9_stage3/3
  filter_in9_stage2/1 -> filter_in9_stage3/2
  filter_in6_stage2/2 -> filter_in6_stage3/3
  filter_in6_stage2/1 -> filter_in6_stage3/2
  filter_in9_stage1/2 -> filter_in9_stage2/3
  filter_in9_stage1/1 -> filter_in9_stage2/2
  filter_in6_stage1/2 -> filter_in6_stage2/3
  filter_in6_stage1/1 -> filter_in6_stage2/2
  coeff_gen_6_9/3(coeff_rev) -> filter_in9_stage1/2
  coeff_gen_6_9/2(coeff) -> filter_in6_stage1/2
  Delay30/1 -> coeff_gen_6_9/1(sync)
  Convert11/1 -> out10/1
  Scale11/1 -> Convert11/1
  Convert10/1 -> out5/1
  Scale10/1 -> Convert10/1
  filter_in10_stage4/1 -> Scale11/1
  filter_in5_stage4/1 -> Scale10/1
  filter_in10_stage3/2 -> filter_in10_stage4/3
  filter_in10_stage3/1 -> filter_in10_stage4/2
  multi_delay_bram_fast_0/36(out35) -> filter_in10_stage4/1
  filter_in5_stage3/2 -> filter_in5_stage4/3
  filter_in5_stage3/1 -> filter_in5_stage4/2
  multi_delay_bram_fast_0/35(out34) -> filter_in5_stage4/1
  filter_in10_stage2/2 -> filter_in10_stage3/3
  filter_in10_stage2/1 -> filter_in10_stage3/2
  filter_in5_stage2/2 -> filter_in5_stage3/3
  filter_in5_stage2/1 -> filter_in5_stage3/2
  filter_in10_stage1/2 -> filter_in10_stage2/3
  filter_in10_stage1/1 -> filter_in10_stage2/2
  filter_in5_stage1/2 -> filter_in5_stage2/3
  filter_in5_stage1/1 -> filter_in5_stage2/2
  coeff_gen_5_10/3(coeff_rev) -> filter_in10_stage1/2
  coeff_gen_5_10/2(coeff) -> filter_in5_stage1/2
  Delay25/1 -> coeff_gen_5_10/1(sync)
  Convert9/1 -> out11/1
  Scale9/1 -> Convert9/1
  Convert8/1 -> out4/1
  Scale8/1 -> Convert8/1
  filter_in11_stage4/1 -> Scale9/1
  filter_in4_stage4/1 -> Scale8/1
  filter_in11_stage3/2 -> filter_in11_stage4/3
  filter_in11_stage3/1 -> filter_in11_stage4/2
  multi_delay_bram_fast_0/30(out29) -> filter_in11_stage4/1
  filter_in4_stage3/2 -> filter_in4_stage4/3
  filter_in4_stage3/1 -> filter_in4_stage4/2
  multi_delay_bram_fast_0/29(out28) -> filter_in4_stage4/1
  filter_in11_stage2/2 -> filter_in11_stage3/3
  filter_in11_stage2/1 -> filter_in11_stage3/2
  filter_in4_stage2/2 -> filter_in4_stage3/3
  filter_in4_stage2/1 -> filter_in4_stage3/2
  filter_in11_stage1/2 -> filter_in11_stage2/3
  filter_in11_stage1/1 -> filter_in11_stage2/2
  filter_in4_stage1/2 -> filter_in4_stage2/3
  filter_in4_stage1/1 -> filter_in4_stage2/2
  coeff_gen_4_11/3(coeff_rev) -> filter_in11_stage1/2
  coeff_gen_4_11/2(coeff) -> filter_in4_stage1/2
  Delay20/1 -> coeff_gen_4_11/1(sync)
  Convert7/1 -> out12/1
  Scale7/1 -> Convert7/1
  Convert6/1 -> out3/1
  Scale6/1 -> Convert6/1
  filter_in12_stage4/1 -> Scale7/1
  filter_in3_stage4/1 -> Scale6/1
  filter_in12_stage3/2 -> filter_in12_stage4/3
  filter_in12_stage3/1 -> filter_in12_stage4/2
  multi_delay_bram_fast_0/24(out23) -> filter_in12_stage4/1
  filter_in3_stage3/2 -> filter_in3_stage4/3
  filter_in3_stage3/1 -> filter_in3_stage4/2
  multi_delay_bram_fast_0/23(out22) -> filter_in3_stage4/1
  filter_in12_stage2/2 -> filter_in12_stage3/3
  filter_in12_stage2/1 -> filter_in12_stage3/2
  filter_in3_stage2/2 -> filter_in3_stage3/3
  filter_in3_stage2/1 -> filter_in3_stage3/2
  filter_in12_stage1/2 -> filter_in12_stage2/3
  filter_in12_stage1/1 -> filter_in12_stage2/2
  filter_in3_stage1/2 -> filter_in3_stage2/3
  filter_in3_stage1/1 -> filter_in3_stage2/2
  coeff_gen_3_12/3(coeff_rev) -> filter_in12_stage1/2
  coeff_gen_3_12/2(coeff) -> filter_in3_stage1/2
  Delay15/1 -> coeff_gen_3_12/1(sync)
  Convert5/1 -> out13/1
  Scale5/1 -> Convert5/1
  Convert4/1 -> out2/1
  Scale4/1 -> Convert4/1
  filter_in13_stage4/1 -> Scale5/1
  filter_in2_stage4/1 -> Scale4/1
  filter_in13_stage3/2 -> filter_in13_stage4/3
  filter_in13_stage3/1 -> filter_in13_stage4/2
  multi_delay_bram_fast_0/18(out17) -> filter_in13_stage4/1
  filter_in2_stage3/2 -> filter_in2_stage4/3
  filter_in2_stage3/1 -> filter_in2_stage4/2
  multi_delay_bram_fast_0/17(out16) -> filter_in2_stage4/1
  filter_in13_stage2/2 -> filter_in13_stage3/3
  filter_in13_stage2/1 -> filter_in13_stage3/2
  filter_in2_stage2/2 -> filter_in2_stage3/3
  filter_in2_stage2/1 -> filter_in2_stage3/2
  filter_in13_stage1/2 -> filter_in13_stage2/3
  filter_in13_stage1/1 -> filter_in13_stage2/2
  filter_in2_stage1/2 -> filter_in2_stage2/3
  filter_in2_stage1/1 -> filter_in2_stage2/2
  coeff_gen_2_13/3(coeff_rev) -> filter_in13_stage1/2
  coeff_gen_2_13/2(coeff) -> filter_in2_stage1/2
  Delay10/1 -> coeff_gen_2_13/1(sync)
  Convert3/1 -> out14/1
  Scale3/1 -> Convert3/1
  Convert2/1 -> out1/1
  Scale2/1 -> Convert2/1
  filter_in14_stage4/1 -> Scale3/1
  filter_in1_stage4/1 -> Scale2/1
  filter_in14_stage3/2 -> filter_in14_stage4/3
  filter_in14_stage3/1 -> filter_in14_stage4/2
  multi_delay_bram_fast_0/12(out11) -> filter_in14_stage4/1
  filter_in1_stage3/2 -> filter_in1_stage4/3
  filter_in1_stage3/1 -> filter_in1_stage4/2
  multi_delay_bram_fast_0/11(out10) -> filter_in1_stage4/1
  filter_in14_stage2/2 -> filter_in14_stage3/3
  filter_in14_stage2/1 -> filter_in14_stage3/2
  filter_in1_stage2/2 -> filter_in1_stage3/3
  filter_in1_stage2/1 -> filter_in1_stage3/2
  filter_in14_stage1/2 -> filter_in14_stage2/3
  filter_in14_stage1/1 -> filter_in14_stage2/2
  filter_in1_stage1/2 -> filter_in1_stage2/3
  filter_in1_stage1/1 -> filter_in1_stage2/2
  coeff_gen_1_14/3(coeff_rev) -> filter_in14_stage1/2
  coeff_gen_1_14/2(coeff) -> filter_in1_stage1/2
  Delay5/1 -> coeff_gen_1_14/1(sync)
  Convert1/1 -> out15/1
  Scale1/1 -> Convert1/1
  Convert/1 -> out0/1
  Scale/1 -> Convert/1
  filter_in15_stage4/1 -> Scale1/1
  filter_in0_stage4/1 -> Scale/1
  filter_in15_stage3/2 -> filter_in15_stage4/3
  filter_in15_stage3/1 -> filter_in15_stage4/2
  multi_delay_bram_fast_0/6(out5) -> filter_in15_stage4/1
  filter_in0_stage3/2 -> filter_in0_stage4/3
  filter_in0_stage3/1 -> filter_in0_stage4/2
  multi_delay_bram_fast_0/5(out4) -> filter_in0_stage4/1
  filter_in15_stage2/2 -> filter_in15_stage3/3
  filter_in15_stage2/1 -> filter_in15_stage3/2
  filter_in0_stage2/2 -> filter_in0_stage3/3
  filter_in0_stage2/1 -> filter_in0_stage3/2
  filter_in15_stage1/2 -> filter_in15_stage2/3
  filter_in15_stage1/1 -> filter_in15_stage2/2
  filter_in0_stage1/2 -> filter_in0_stage2/3
  filter_in0_stage1/1 -> filter_in0_stage2/2
  coeff_gen_0_15/3(coeff_rev) -> filter_in15_stage1/2
  coeff_gen_0_15/2(coeff) -> filter_in0_stage1/2
  Delay/1 -> coeff_gen_0_15/1(sync)
